simulink model "CdsnProbltyMgr"  (R2022b)
settings: Solver=FixedStepDiscrete  StartTime=0.0  StopTime=10.0  FixedStep=0.05  RelTol=1e-3
(part 1 of 2)
settings Model: Creator=blue.ge  LastModifiedBy=blue.ge
settings DataIOCC: SaveFormat=StructureWithTime
settings OptimizationCC: DefaultParameterBehavior=Inlined  EnhancedBackFolding=on  PassReuseOutputArgsAs=Individual arguments  ZeroExternalMemoryAtStartup=off  ZeroInternalMemoryAtStartup=off  OptimizeBlockOrder=speed  DifferentSizesBufferReuse=on  OptimizationCustomize=off  EfficientTunableParamExpr=on
settings DebuggingCC: ParameterTunabilityLossMsg=error
settings ModelReferenceCC: ModelReferenceNumInstancesAllowed=Single
settings SFSimCC: MATLABDynamicMemAlloc=off
settings RTWCC: Description=AUTOSAR  SystemTargetFile=autosar.tlc  GenCodeOnly=on  TemplateMakefile=ert_default_tmf
settings CodeAppCC: ForceParamTrailComments=on  IgnoreCustomStorageClasses=off  OperatorAnnotations=on  SimulinkDataObjDesc=on  SFDataObjDesc=on  InsertBlockDesc=on
settings STFCustomTargetCC: TargetLangStandard=C99 (ISO)  MultiwordLength=256  ParMdlRefBuildCompliant=on  CompOptLevelCompliant=on  IncludeMdlTerminateFcn=off  CombineOutputUpdateFcns=on  SuppressErrorStatus=on  ERTCustomFileBanners=on  SupportNonFinite=off  SupportComplex=off  SupportContinuousTime=off  SupportNonInlinedSFcns=off  RemoveResetFunc=on  MATLABClassNameForMDSCustomization=Simulink.SoftwareTarget.ERTCustomization  AutosarCompliant=on  UseToolchainInfoCompliant=on  LookupTableObjectStructAxisOrder=2,1,3,4,...  LUTObjectStructOrderEvenSpacing=Size,Table,Breakpoints  SystemTargetFile=autosar.tlc
settings ERTTargetCC: TargetLangStandard=C99 (ISO)  ConcurrentExecutionCompliant=off  IncludeMdlTerminateFcn=off  SuppressErrorStatus=on  SupportNonFinite=off  SupportComplex=off  ModelStepFunctionPrototypeControlCompliant=off  CPPClassGenCompliant=off  AutosarCompliant=on  LookupTableObjectStructAxisOrder=2,1,3,4,...  LUTObjectStructOrderEvenSpacing=Size,Table,Breakpoints  InlinedParameterPlacement=NonHierarchical  ERTCustomFileTemplate=example_file_process.tlc

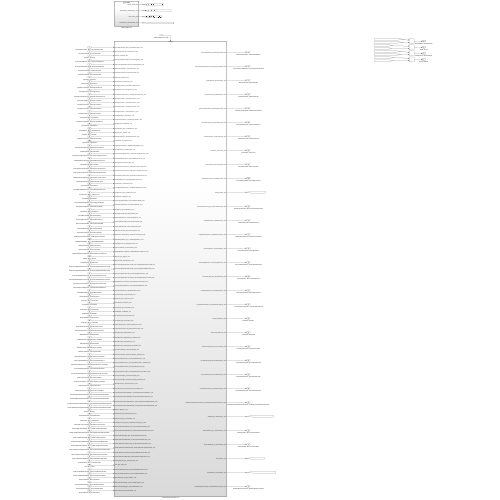
[thumbnail: Simulink's stored preview of the top-level diagram]
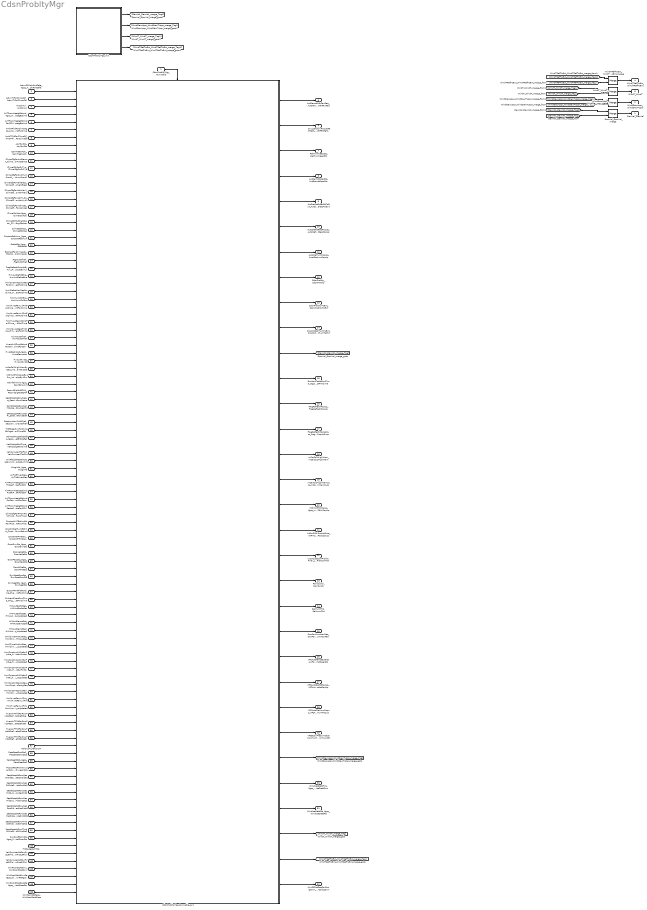
== system CdsnProbltyMgr ==
  CdsnProbltyMgr_Runnable : Inport  SampleTime=0.05  OutputFunctionCall=on
  ActvnOfWshrFrntSafe_Ngcc_ActvnOfWshrFrntSafe : Inport  Port=2  OutDataTypeStr=Enum: OnOffCrit1  PortDimensions=1
  ActvnTiForClimaCtrl_ActvnTiForClimaCtrl : Inport  Port=3  OutDataTypeStr=Ti33  PortDimensions=1
  AirCdnAvl_AirCdnAvl : Inport  Port=4  OutDataTypeStr=Boolean  PortDimensions=1
  AirTFromHeatgEstimd_Ngcc_AirTFromHeatgEstimd : Inport  Port=5  OutDataTypeStr=T30  PortDimensions=1
  AirTFromHeatgEstimdForCCM_AirTFromHeatgEstimd : Inport  Port=6  OutDataTypeStr=T30  PortDimensions=1
  AmbTFildForClimaNgcc_AmbTFildForClima : Inport  Port=7  OutDataTypeStr=T30  PortDimensions=1
  AmbTFildForClimaQf_AmbTFildForClimaQf : Inport  Port=8  OutDataTypeStr=Enum: GenQf1  PortDimensions=1
  AsyFanSts_AsyFanSts : Inport  Port=9  OutDataTypeStr=Enum: OnOff1  PortDimensions=1
  CamHeatrActv_CamHeatrActv : Inport  Port=10  OutDataTypeStr=Enum: OnOff1  PortDimensions=1
  ClimaCfgForAutDemst_ClimaCfgForAutDemst : Inport  Port=11  OutDataTypeStr=Boolean  PortDimensions=1
  ClimaCfgForFlcTyp_ClimaCfgForFlcTyp : Inport  Port=12  OutDataTypeStr=Enum: CarCfgForFlcTyp  PortDimensions=1
  ClimaCfgForSnsrHumPrsnt1_ClimaCfgForSnsrHumPrsnt1 : Inport  Port=13  OutDataTypeStr=Boolean  PortDimensions=1
  ClimaCfgForVehElec1_ClimaCfgForVehElec1 : Inport  Port=14  OutDataTypeStr=Boolean  PortDimensions=1
  ClimaCfgForVehHev1_ClimaCfgForVehHev1 : Inport  Port=15  OutDataTypeStr=Boolean  PortDimensions=1
  ClimaCfgForVehHyb1_ClimaCfgForVehHyb1 : Inport  Port=16  OutDataTypeStr=Boolean  PortDimensions=1
  ClimaCfgForVehIce1_ClimaCfgForVehIce1 : Inport  Port=17  OutDataTypeStr=Boolean  PortDimensions=1
  ClimaCtrlActvNgcc_ClimaCtrlActv : Inport  Port=18  OutDataTypeStr=Boolean  PortDimensions=1
  ClimaCtrlForEngStbActv_ClimaCtrlForEngStbActv : Inport  Port=19  OutDataTypeStr=Boolean  PortDimensions=1
  ClimaStStrtUp_ClimaStStrtUp : Inport  Port=20  OutDataTypeStr=Enum: ClimaStStrtUp  PortDimensions=1
  CmptmtRelHum_Ngcc_CmptmtRelHum : Inport  Port=21  OutDataTypeStr=Bus: CmptmRelHum  PortDimensions=1  BusOutputAsStruct=on
  DefrstSts_Ngcc_DefrstSts : Inport  Port=22  OutDataTypeStr=Bus: DefrstSts  PortDimensions=1  BusOutputAsStruct=on
  EcoModForClimaActv_EcoModForClimaActv : Inport  Port=23  OutDataTypeStr=Enum: OnOff1  PortDimensions=1
  FogOutOfVeh_FogOutOfVeh : Inport  Port=24  OutDataTypeStr=Bus: FogOutOfVeh  PortDimensions=1  BusOutputAsStruct=on
  FragRefreshPopUpByAux_FragRefreshPopUpByAux : Inport  Port=25  OutDataTypeStr=Enum: OnOff1  PortDimensions=1
  HmiAutDefrstEna_HmiAutDefrstEna : Inport  Port=26  OutDataTypeStr=Enum: OnOff1  PortDimensions=1
  HmiCmptmtCoolgReqForClima_HmiCmptmtCoolgReqForClima : Inport  Port=27  OutDataTypeStr=Enum: HmiCmptmtCoolgReq  PortDimensions=1
  HmiDefrstMaxReqForClima_HmiDefrstMaxReqForClima : Inport  Port=28  OutDataTypeStr=Enum: ActrReq  PortDimensions=1
  HmiHumCtrlEna_HmiHumCtrlEna : Inport  Port=29  OutDataTypeStr=Enum: OnOff1  PortDimensions=1
  HmiHvacFanLvlFrntForClima_HmiHvacFanLvlFrntForClima : Inport  Port=30  OutDataTypeStr=Enum: HmiHvacFanLvl  PortDimensions=1
  HmiHvacFanLvlFrntForClima_HmiHvacFanLvlFrntRiForClima : Inport  Port=31  OutDataTypeStr=Enum: HmiHvacFanLvl  PortDimensions=1
  HmiHvacRecircCmdForClima_HmiHvacRecircCmdForClima : Inport  Port=32  OutDataTypeStr=Enum: HmiHvacRecircCmd  PortDimensions=1
  HmiMaxACReqForClima_HmiMaxACReqForClima : Inport  Port=33  OutDataTypeStr=Enum: OnOff1  PortDimensions=1
  HumOutOfVeh_HumOutOfVeh : Inport  Port=34  OutDataTypeStr=Bus: HumOutOfVeh  PortDimensions=1  BusOutputAsStruct=on
  HvacAirMFlowEstimdForCtrlr_HvacAirMFlowEstimdForCtrlr : Inport  Port=35  OutDataTypeStr=AirFlow30  PortDimensions=1
  HvacRecircAct_Ngcc_HvacRecircAct : Inport  Port=36  OutDataTypeStr=Perc4  PortDimensions=1
  HvSysRlySts_HvSysRlySts : Inport  Port=37  OutDataTypeStr=Bus: HvSysRlySts  PortDimensions=1  BusOutputAsStruct=on
  InCarCo2HighWarnByCDS_InCarCo2HighWarnByCDS : Inport  Port=38  OutDataTypeStr=Enum: Co2Lvl  PortDimensions=1
  IntPm25HiPopUpByAPm_IntPm25HiPopUpByAPm : Inport  Port=39  OutDataTypeStr=Enum: IntPmHiPopUp  PortDimensions=1
  RainfallAmnt_Ngcc_RainfallAmnt : Inport  Port=40  OutDataTypeStr=Enum: AmntSnsr  PortDimensions=1
  ResrvdSigForECM4_ResrvdSigForECM4 : Inport  Port=41  OutDataTypeStr=BattSnsrChksTbl  PortDimensions=1
  SeatOccptAtRowSecLe_SeatOccptAtRowSecLe : Inport  Port=42  OutDataTypeStr=Enum: PassSeatSts1  PortDimensions=1
  SeatOccptAtRowSecMid_SeatOccptAtRowSecMid : Inport  Port=43  OutDataTypeStr=Enum: PassSeatSts1  PortDimensions=1
  SeatOccptAtRowSecRi_SeatOccptAtRowSecRi : Inport  Port=44  OutDataTypeStr=Enum: PassSeatSts1  PortDimensions=1
  SessionActvOutOfVeh_SessionActvOutOfVeh : Inport  Port=45  OutDataTypeStr=Enum: NoYes1  PortDimensions=1
  TiOfDeactvnForClimaCtrlNgcc_TiOfDeactvnForClimaCtrl : Inport  Port=46  OutDataTypeStr=Ti33  PortDimensions=1
  VehModMngtGlbSafe1_Ngcc_VehModMngtGlbSafe1 : Inport  Port=47  OutDataTypeStr=Bus: VehModMngtGlbSafe1  PortDimensions=1  BusOutputAsStruct=on
  VehSpdLgtForClima_VehSpdLgtForClima : Inport  Port=48  OutDataTypeStr=Bus: VehSpdLgtForClima  PortDimensions=1  BusOutputAsStruct=on
  VehSunLoadTotFild_VehSunLoadTotFild : Inport  Port=49  OutDataTypeStr=SunLoad30  PortDimensions=1
  WinRfClsdReqForCoolgDwnInt_WinRfClsdReqForCoolgDwnInt : Inport  Port=50  OutDataTypeStr=Enum: Warn2  PortDimensions=1
  WipgInfo_Ngcc_WipgInfo : Inport  Port=51  OutDataTypeStr=Bus: WipgInfo  PortDimensions=1  BusOutputAsStruct=on
  AirTAftHvacDes_AirTAftHvacDes : Inport  Port=52  OutDataTypeStr=T30  PortDimensions=1
  AirTFromHeatgEstimdFirstLeForCCM_AirTFromHeatgEstimdFirstLeForCCM : Inport  Port=53  OutDataTypeStr=T30  PortDimensions=1
  AirTFromHeatgEstimdFirstRiForCCM_AirTFromHeatgEstimdFirstRiForCCM : Inport  Port=54  OutDataTypeStr=T30  PortDimensions=1
  AirTFromHeatgEstimdForCdsn_AirTFromHeatgEstimdForCdsn : Inport  Port=55  OutDataTypeStr=T30  PortDimensions=1
  AirTFromHeatgEstimdSecLeForCCM_AirTFromHeatgEstimdSecLeForCCM : Inport  Port=56  OutDataTypeStr=T30  PortDimensions=1
  ClimaCfgForPTCInFrontHVAC_ClimaCfgForPTCInFrontHVAC : Inport  Port=57  OutDataTypeStr=Enum: FrontHeaterExchanger  PortDimensions=1
  CmptmtAirTEstimdAtRowFirst_CmptmtAirTEstimdAtRowFirst : Inport  Port=58  OutDataTypeStr=T30  PortDimensions=1
  CmptmtRelHumEstimd_CmptmtRelHumEstimd : Inport  Port=59  OutDataTypeStr=Perc7  PortDimensions=1
  CmptmtTFrntRaw_CmptmtTFrntRaw : Inport  Port=60  OutDataTypeStr=Bus: CmptmtTFrnt  PortDimensions=1  BusOutputAsStruct=on
  DoorDrvrSts_Ngcc_DoorDrvrSts : Inport  Port=61  OutDataTypeStr=Enum: DoorSts2  PortDimensions=1
  DoorLeReSts_DoorLeReSts : Inport  Port=62  OutDataTypeStr=Enum: DoorSts2  PortDimensions=1
  DoorPassSts_Ngcc_DoorPassSts : Inport  Port=63  OutDataTypeStr=Enum: DoorSts2  PortDimensions=1
  DoorRiReSts_DoorRiReSts : Inport  Port=64  OutDataTypeStr=Enum: DoorSts2  PortDimensions=1
  DrvrSeatSnsrSts_DrvrSeatSnsrSts : Inport  Port=65  OutDataTypeStr=Enum: OkNokDi  PortDimensions=1
  DrvrSeatSts_Ngcc_DrvrSeatSts : Inport  Port=66  OutDataTypeStr=Enum: OccptPresSt1  PortDimensions=1
  EvaprTFrntFildForClima_EvaprTFrntFildForClima : Inport  Port=67  OutDataTypeStr=T30  PortDimensions=1
  ExtVentToastFrmClima_Prkg_ExtVentToastFrmClima : Inport  Port=68  OutDataTypeStr=Enum: ExtVentToastFrmClimaSts  PortDimensions=1
  HMIAutDefrstSet_HMIAutDefrstSet : Inport  Port=69  OutDataTypeStr=Enum: OnOff1  PortDimensions=1
  HMIAutDefrstSet_HMIAutDefrstSet_IsUpdated : Inport  Port=70  OutDataTypeStr=boolean  PortDimensions=1
  HMIAutDemstSet_HMIAutDemstSet : Inport  Port=71  OutDataTypeStr=Enum: OnOff1  PortDimensions=1
  HMIAutDemstSet_HMIAutDemstSet_IsUpdated : Inport  Port=72  OutDataTypeStr=boolean  PortDimensions=1
  HmiClimaFrntAutReq_HmiClimaFrntAutReq : Inport  Port=73  OutDataTypeStr=Enum: OnOff1  PortDimensions=1
  HmiClimaFrntAutReq_HmiClimaFrntAutReq_IsUpdated : Inport  Port=74  OutDataTypeStr=boolean  PortDimensions=1
  HmiCmptmtAirDistbnFrntLe_HmiCmptmtAirDistbnFrntLe : Inport  Port=75  OutDataTypeStr=Enum: HmiCmptmtAirDistbnFrnt  PortDimensions=1
  HmiCmptmtAirDistbnFrntLe_HmiCmptmtAirDistbnFrntLe_IsUpdated : Inport  Port=76  OutDataTypeStr=boolean  PortDimensions=1
  HmiCmptmtAirDistbnFrntRi_HmiCmptmtAirDistbnFrntRi : Inport  Port=77  OutDataTypeStr=Enum: HmiCmptmtAirDistbnFrnt  PortDimensions=1
  HmiCmptmtAirDistbnFrntRi_HmiCmptmtAirDistbnFrntRi_IsUpdated : Inport  Port=78  OutDataTypeStr=boolean  PortDimensions=1
  HmiCmptmtCoolgReq_HmiCmptmtCoolgReq : Inport  Port=79  OutDataTypeStr=Enum: HmiCmptmtCoolgReq  PortDimensions=1
  HmiCmptmtCoolgReq_HmiCmptmtCoolgReq_IsUpdated : Inport  Port=80  OutDataTypeStr=boolean  PortDimensions=1
  HmiHvacFanLvlFrnt_HmiHvacFanLvlFrnt : Inport  Port=81  OutDataTypeStr=Enum: HmiHvacFanLvl  PortDimensions=1
  HmiHvacFanLvlFrnt_HmiHvacFanLvlFrnt_IsUpdated : Inport  Port=82  OutDataTypeStr=boolean  PortDimensions=1
  HvacAirTFildForRowFirstAtDefrstLe_HvacAirTFildForRowFirstAtDefrstLe : Inport  Port=83  OutDataTypeStr=T30  PortDimensions=1
  HvacAirTFildForRowFirstAtDefrstRi_HvacAirTFildForRowFirstAtDefrstRi : Inport  Port=84  OutDataTypeStr=T30  PortDimensions=1
  HvacAirTFildForRowFirstAtDefrstSideLe_HvacAirTFildForRowFirstAtDefrstSideLe : Inport  Port=85  OutDataTypeStr=T30  PortDimensions=1
  HvacAirTFildForRowFirstAtDefrstSideRi_HvacAirTFildForRowFirstAtDefrstSideRi : Inport  Port=86  OutDataTypeStr=T30  PortDimensions=1
  MtrlSnsrT_MtrlSnsrT : Inport  Port=87  OutDataTypeStr=Bus: MtrlSnsrT  PortDimensions=1  BusOutputAsStruct=on
  PassSeatSnsrSts2_PassSeatSnsrSts2 : Inport  Port=88  OutDataTypeStr=Enum: OkNokDi  PortDimensions=1
  PassSeatSts2_Ngcc_PassSeatSts2 : Inport  Port=89  OutDataTypeStr=Enum: OccptPresSt1  PortDimensions=1
  PopUpReqFromOxygenSply_PopUpReqFromOxygenSply : Inport  Port=90  OutDataTypeStr=Enum: OxygenSplyPopUpReq  PortDimensions=1
  SeatOccptAtRowSecDrSide2_Ngcc_SeatOccptAtRowSecDrSide2 : Inport  Port=91  OutDataTypeStr=Enum: OccptPresSt1  PortDimensions=1
  SeatOccptAtRowSecDrSideSnsSts2_SeatOccptAtRowSecDrSideSnsSts2 : Inport  Port=92  OutDataTypeStr=Enum: OkNokDi  PortDimensions=1
  SeatOccptAtRowSecMid2_Ngcc_SeatOccptAtRowSecMid2 : Inport  Port=93  OutDataTypeStr=Enum: OccptPresSt1  PortDimensions=1
  SeatOccptAtRowSecMidSnsSts2_SeatOccptAtRowSecMidSnsSts2 : Inport  Port=94  OutDataTypeStr=Enum: OkNokDi  PortDimensions=1
  SeatOccptAtRowSecPasSide2_Ngcc_SeatOccptAtRowSecPasSide2 : Inport  Port=95  OutDataTypeStr=Enum: OccptPresSt1  PortDimensions=1
  SeatOccptAtRowSecPasSideSnsSts2_SeatOccptAtRowSecPasSideSnsSts2 : Inport  Port=96  OutDataTypeStr=Enum: OkNokDi  PortDimensions=1
  SeatOccptAtRowThrdLeSnsSts2_SeatOccptAtRowThrdLeSnsSts2 : Inport  Port=97  OutDataTypeStr=Enum: OkNokDi  PortDimensions=1
  SeatOccptAtRowThrdRiSnsSts2_SeatOccptAtRowThrdRiSnsSts2 : Inport  Port=98  OutDataTypeStr=Enum: OkNokDi  PortDimensions=1
  SunRoofPosnSts_Ngcc_SunRoofPosnSts : Inport  Port=99  OutDataTypeStr=Enum: WinAndRoofAndCurtPosnTyp  PortDimensions=1
  TrSts_Ngcc_TrSts : Inport  Port=100  OutDataTypeStr=Enum: DoorSts2  PortDimensions=1
  VehSunLoadAtRowFirstLeFild_VehSunLoadAtRowFirstLeFild : Inport  Port=101  OutDataTypeStr=SunLoad30  PortDimensions=1
  VehSunLoadAtRowFirstRiFild_VehSunLoadAtRowFirstRiFild : Inport  Port=102  OutDataTypeStr=SunLoad30  PortDimensions=1
  WinPosnStsAtDrvr_WinPosnStsAtDrvr : Inport  Port=103  OutDataTypeStr=Enum: WinAndRoofAndCurtPosnTyp  PortDimensions=1
  WinPosnStsAtDrvrReNgcc_WinPosnStsAtDrvrReNgcc : Inport  Port=104  OutDataTypeStr=Enum: WinAndRoofAndCurtPosnTyp  PortDimensions=1
  WinPosnStsAtPassReNgcc_WinPosnStsAtPassRe : Inport  Port=105  OutDataTypeStr=Enum: WinAndRoofAndCurtPosnTyp  PortDimensions=1
  WinPosnStsPass_WinPosnStsAtPass : Inport  Port=106  OutDataTypeStr=Enum: WinAndRoofAndCurtPosnTyp  PortDimensions=1
  CdsnProbltyMgr_Init : Subsystem
  CdsnProbltyMgr_Runnable_sys : Subsystem
  DewVal_DewVal_merge : Merge
  WindDewUppr_WindDewTUppr_merge : Merge
  WindTRefToExt_WindTRefToExt_merge : Merge
  WindT_WindT_merge : Merge
  DewVal_DewVal : Outport  OutDataTypeStr=Bus: AirDewMeasValReq  PortDimensions=1  BusOutputAsStruct=on
  WindDewUppr_WindDewTUppr : Outport  Port=2  OutDataTypeStr=Bus: WindDewTUppr  PortDimensions=1  BusOutputAsStruct=on
  WindT_WindT : Outport  Port=3  OutDataTypeStr=Bus: WindT  PortDimensions=1  BusOutputAsStruct=on
  WindTRefToExt_WindTRefToExt : Outport  Port=4  OutDataTypeStr=Bus: WindT  PortDimensions=1  BusOutputAsStruct=on
  AutDemstWthFanReq_AutDemstWthFanReq : Outport  Port=5  OutDataTypeStr=UInt8  PortDimensions=1
  OxygenSplyPopUpReqNgcc_OxygenSplyPopUpReqNgcc : Outport  Port=6  OutDataTypeStr=Enum: OxygenSplyPopUpReq  PortDimensions=1
  RelHumKeepSts_RelHumKeepSts : Outport  Port=7  OutDataTypeStr=Enum: RelHumKeepSts  PortDimensions=1
  AutDemstOperSts_AutDemstOperSts : Outport  Port=8  OutDataTypeStr=Enum: OnOff1  PortDimensions=1
  AutDemstOperStsToExit_AutDemstOperStsToExit : Outport  Port=9  OutDataTypeStr=Enum: OnOff1  PortDimensions=1
  AutoDefrstReqPopUp_AutoDefrstReqPopUp : Outport  Port=10  OutDataTypeStr=Enum: OnOff1  PortDimensions=1
  AutoDeHumPopUp_AutoDeHumPopUp : Outport  Port=11  OutDataTypeStr=Enum: ReqSts1  PortDimensions=1
  CdsnProblty_CdsnProblty : Outport  Port=12  OutDataTypeStr=Fac30  PortDimensions=1
  CdsnProbltyToExt_CdsnProbltyToExt : Outport  Port=13  OutDataTypeStr=Fac30  PortDimensions=1
  CmptmtRelHumToExt_CmptmtRelHumToExt : Outport  Port=14  OutDataTypeStr=Bus: CmptmtRelHumToExt  PortDimensions=1  BusOutputAsStruct=on
  ExtVentToastFrmClima_Ngcc_ExtVentToastFrmClima : Outport  Port=15  OutDataTypeStr=Enum: ExtVentToastFrmClimaSts  PortDimensions=1
  FragRefreshPopUp_FragRefreshPopUp : Outport  Port=16  OutDataTypeStr=Enum: OnOff1  PortDimensions=1
  FragRefreshPopUpSucs_FragRefreshPopUpSucs : Outport  Port=17  OutDataTypeStr=Boolean  PortDimensions=1
  InCarCo2HighWarn_InCarCo2HighWarn : Outport  Port=18  OutDataTypeStr=Enum: Co2Lvl  PortDimensions=1
  InCarCo2HighWarnSucs_InCarCo2HighWarnSucs : Outport  Port=19  OutDataTypeStr=Boolean  PortDimensions=1
  IntPm25HiPopUp_Ngcc_IntPm25HiPopUp : Outport  Port=20  OutDataTypeStr=Enum: IntPmHiPopUp  PortDimensions=1
  IntPm25HiPopUpSucs_IntPm25HiPopUpSucs : Outport  Port=21  OutDataTypeStr=Boolean  PortDimensions=1
  LoopClsdCdsnProbltyFinal_LoopClsdCdsnProbltyFinal : Outport  Port=22  OutDataTypeStr=Fac30  PortDimensions=1
  RainSybol_RainSybol : Outport  Port=23  OutDataTypeStr=Boolean  PortDimensions=1
  RelHumFild_RelHumFild : Outport  Port=24  OutDataTypeStr=Perc7  PortDimensions=1
  SnsrForHumActvReq_SnsrForHumActvReq : Outport  Port=25  OutDataTypeStr=Enum: OnOff1  PortDimensions=1
  VFCAutDemstOperSts_VFCAutDemstOperSts : Outport  Port=26  OutDataTypeStr=Boolean  PortDimensions=1
  VFCAutoDefrstPopUp_VFCAutoDefrstPopUp : Outport  Port=27  OutDataTypeStr=Boolean  PortDimensions=1
  VFCAutoDeHumPopup_VFCAutoDeHumPopup : Outport  Port=28  OutDataTypeStr=Boolean  PortDimensions=1
  VFCBodyPreClimatisationHmiHumCtrl_VFCBodyPreClimatisationHmiHumCtrl : Outport  Port=29  OutDataTypeStr=Boolean  PortDimensions=1
  WindIceDetdFrnt_Ngcc_WindIceDetdFrnt : Outport  Port=30  OutDataTypeStr=Enum: OnOff1  PortDimensions=1
  WindIceDetdRe_Ngcc_WindIceDetdRe : Outport  Port=31  OutDataTypeStr=Enum: OnOff1  PortDimensions=1
  WinRfClsdReqForCoolgDwn_WinRfClsdReqForCoolgDwn : Outport  Port=32  OutDataTypeStr=Enum: Warn2  PortDimensions=1
  wires:
  ActvnOfWshrFrntSafe_Ngcc_ActvnOfWshrFrntSafe/1 -> CdsnProbltyMgr_Runnable_sys/1(ActvnOfWshrFrntSafe_Ngcc_ActvnOfWshrFrntSafe_read)
  ActvnTiForClimaCtrl_ActvnTiForClimaCtrl/1 -> CdsnProbltyMgr_Runnable_sys/2(ActvnTiForClimaCtrl_ActvnTiForClimaCtrl_read)
  AirCdnAvl_AirCdnAvl/1 -> CdsnProbltyMgr_Runnable_sys/3(AirCdnAvl_AirCdnAvl_read)
  AirTFromHeatgEstimd_Ngcc_AirTFromHeatgEstimd/1 -> CdsnProbltyMgr_Runnable_sys/4(AirTFromHeatgEstimd_Ngcc_AirTFromHeatgEstimd_read)
  AirTFromHeatgEstimdForCCM_AirTFromHeatgEstimd/1 -> CdsnProbltyMgr_Runnable_sys/5(AirTFromHeatgEstimdForCCM_AirTFromHeatgEstimd_read)
  AmbTFildForClimaNgcc_AmbTFildForClima/1 -> CdsnProbltyMgr_Runnable_sys/6(AmbTFildForClimaNgcc_AmbTFildForClima_read)
  AmbTFildForClimaQf_AmbTFildForClimaQf/1 -> CdsnProbltyMgr_Runnable_sys/7(AmbTFildForClimaQf_AmbTFildForClimaQf_read)
  AsyFanSts_AsyFanSts/1 -> CdsnProbltyMgr_Runnable_sys/8(AsyFanSts_AsyFanSts_read)
  CamHeatrActv_CamHeatrActv/1 -> CdsnProbltyMgr_Runnable_sys/9(CamHeatrActv_CamHeatrActv_read)
  ClimaCfgForAutDemst_ClimaCfgForAutDemst/1 -> CdsnProbltyMgr_Runnable_sys/10(ClimaCfgForAutDemst_ClimaCfgForAutDemst_read)
  ClimaCfgForFlcTyp_ClimaCfgForFlcTyp/1 -> CdsnProbltyMgr_Runnable_sys/11(ClimaCfgForFlcTyp_ClimaCfgForFlcTyp_read)
  ClimaCfgForSnsrHumPrsnt1_ClimaCfgForSnsrHumPrsnt1/1 -> CdsnProbltyMgr_Runnable_sys/12(ClimaCfgForSnsrHumPrsnt1_ClimaCfgForSnsrHumPrsnt1_read)
  ClimaCfgForVehElec1_ClimaCfgForVehElec1/1 -> CdsnProbltyMgr_Runnable_sys/13(ClimaCfgForVehElec1_ClimaCfgForVehElec1_read)
  ClimaCfgForVehHev1_ClimaCfgForVehHev1/1 -> CdsnProbltyMgr_Runnable_sys/14(ClimaCfgForVehHev1_ClimaCfgForVehHev1_read)
  ClimaCfgForVehHyb1_ClimaCfgForVehHyb1/1 -> CdsnProbltyMgr_Runnable_sys/15(ClimaCfgForVehHyb1_ClimaCfgForVehHyb1_read)
  ClimaCfgForVehIce1_ClimaCfgForVehIce1/1 -> CdsnProbltyMgr_Runnable_sys/16(ClimaCfgForVehIce1_ClimaCfgForVehIce1_read)
  ClimaCtrlActvNgcc_ClimaCtrlActv/1 -> CdsnProbltyMgr_Runnable_sys/17(ClimaCtrlActvNgcc_ClimaCtrlActv_read)
  ClimaCtrlForEngStbActv_ClimaCtrlForEngStbActv/1 -> CdsnProbltyMgr_Runnable_sys/18(ClimaCtrlForEngStbActv_ClimaCtrlForEngStbActv_read)
  ClimaStStrtUp_ClimaStStrtUp/1 -> CdsnProbltyMgr_Runnable_sys/19(ClimaStStrtUp_ClimaStStrtUp_read)
  CmptmtRelHum_Ngcc_CmptmtRelHum/1 -> CdsnProbltyMgr_Runnable_sys/20(CmptmtRelHum_Ngcc_CmptmtRelHum_read)
  DefrstSts_Ngcc_DefrstSts/1 -> CdsnProbltyMgr_Runnable_sys/21(DefrstSts_Ngcc_DefrstSts_read)
  EcoModForClimaActv_EcoModForClimaActv/1 -> CdsnProbltyMgr_Runnable_sys/22(EcoModForClimaActv_EcoModForClimaActv_read)
  FogOutOfVeh_FogOutOfVeh/1 -> CdsnProbltyMgr_Runnable_sys/23(FogOutOfVeh_FogOutOfVeh_read)
  FragRefreshPopUpByAux_FragRefreshPopUpByAux/1 -> CdsnProbltyMgr_Runnable_sys/24(FragRefreshPopUpByAux_FragRefreshPopUpByAux_read)
  HmiAutDefrstEna_HmiAutDefrstEna/1 -> CdsnProbltyMgr_Runnable_sys/25(HmiAutDefrstEna_HmiAutDefrstEna_read)
  HmiCmptmtCoolgReqForClima_HmiCmptmtCoolgReqForClima/1 -> CdsnProbltyMgr_Runnable_sys/26(HmiCmptmtCoolgReqForClima_HmiCmptmtCoolgReqForClima_read)
  HmiDefrstMaxReqForClima_HmiDefrstMaxReqForClima/1 -> CdsnProbltyMgr_Runnable_sys/27(HmiDefrstMaxReqForClima_HmiDefrstMaxReqForClima_read)
  HmiHumCtrlEna_HmiHumCtrlEna/1 -> CdsnProbltyMgr_Runnable_sys/28(HmiHumCtrlEna_HmiHumCtrlEna_read)
  HmiHvacFanLvlFrntForClima_HmiHvacFanLvlFrntForClima/1 -> CdsnProbltyMgr_Runnable_sys/29(HmiHvacFanLvlFrntForClima_HmiHvacFanLvlFrntForClima_read)
  HmiHvacFanLvlFrntForClima_HmiHvacFanLvlFrntRiForClima/1 -> CdsnProbltyMgr_Runnable_sys/30(HmiHvacFanLvlFrntForClima_HmiHvacFanLvlFrntRiForClima_read)
  HmiHvacRecircCmdForClima_HmiHvacRecircCmdForClima/1 -> CdsnProbltyMgr_Runnable_sys/31(HmiHvacRecircCmdForClima_HmiHvacRecircCmdForClima_read)
  HmiMaxACReqForClima_HmiMaxACReqForClima/1 -> CdsnProbltyMgr_Runnable_sys/32(HmiMaxACReqForClima_HmiMaxACReqForClima_read)
  HumOutOfVeh_HumOutOfVeh/1 -> CdsnProbltyMgr_Runnable_sys/33(HumOutOfVeh_HumOutOfVeh_read)
  HvacAirMFlowEstimdForCtrlr_HvacAirMFlowEstimdForCtrlr/1 -> CdsnProbltyMgr_Runnable_sys/34(HvacAirMFlowEstimdForCtrlr_HvacAirMFlowEstimdForCtrlr_read)
  HvacRecircAct_Ngcc_HvacRecircAct/1 -> CdsnProbltyMgr_Runnable_sys/35(HvacRecircAct_Ngcc_HvacRecircAct_read)
  HvSysRlySts_HvSysRlySts/1 -> CdsnProbltyMgr_Runnable_sys/36(HvSysRlySts_HvSysRlySts_read)
  InCarCo2HighWarnByCDS_InCarCo2HighWarnByCDS/1 -> CdsnProbltyMgr_Runnable_sys/37(InCarCo2HighWarnByCDS_InCarCo2HighWarnByCDS_read)
  IntPm25HiPopUpByAPm_IntPm25HiPopUpByAPm/1 -> CdsnProbltyMgr_Runnable_sys/38(IntPm25HiPopUpByAPm_IntPm25HiPopUpByAPm_read)
  RainfallAmnt_Ngcc_RainfallAmnt/1 -> CdsnProbltyMgr_Runnable_sys/39(RainfallAmnt_Ngcc_RainfallAmnt_read)
  ResrvdSigForECM4_ResrvdSigForECM4/1 -> CdsnProbltyMgr_Runnable_sys/40(ResrvdSigForECM4_ResrvdSigForECM4_read)
  SeatOccptAtRowSecLe_SeatOccptAtRowSecLe/1 -> CdsnProbltyMgr_Runnable_sys/41(SeatOccptAtRowSecLe_SeatOccptAtRowSecLe_read)
  SeatOccptAtRowSecMid_SeatOccptAtRowSecMid/1 -> CdsnProbltyMgr_Runnable_sys/42(SeatOccptAtRowSecMid_SeatOccptAtRowSecMid_read)
  SeatOccptAtRowSecRi_SeatOccptAtRowSecRi/1 -> CdsnProbltyMgr_Runnable_sys/43(SeatOccptAtRowSecRi_SeatOccptAtRowSecRi_read)
  SessionActvOutOfVeh_SessionActvOutOfVeh/1 -> CdsnProbltyMgr_Runnable_sys/44(SessionActvOutOfVeh_SessionActvOutOfVeh_read)
  TiOfDeactvnForClimaCtrlNgcc_TiOfDeactvnForClimaCtrl/1 -> CdsnProbltyMgr_Runnable_sys/45(TiOfDeactvnForClimaCtrlNgcc_TiOfDeactvnForClimaCtrl_read)
  VehModMngtGlbSafe1_Ngcc_VehModMngtGlbSafe1/1 -> CdsnProbltyMgr_Runnable_sys/46(VehModMngtGlbSafe1_Ngcc_VehModMngtGlbSafe1_read)
  VehSpdLgtForClima_VehSpdLgtForClima/1 -> CdsnProbltyMgr_Runnable_sys/47(VehSpdLgtForClima_VehSpdLgtForClima_read)
  VehSunLoadTotFild_VehSunLoadTotFild/1 -> CdsnProbltyMgr_Runnable_sys/48(VehSunLoadTotFild_VehSunLoadTotFild_read)
  WinRfClsdReqForCoolgDwnInt_WinRfClsdReqForCoolgDwnInt/1 -> CdsnProbltyMgr_Runnable_sys/49(WinRfClsdReqForCoolgDwnInt_WinRfClsdReqForCoolgDwnInt_read)
  WipgInfo_Ngcc_WipgInfo/1 -> CdsnProbltyMgr_Runnable_sys/50(WipgInfo_Ngcc_WipgInfo_read)
  AirTAftHvacDes_AirTAftHvacDes/1 -> CdsnProbltyMgr_Runnable_sys/51(AirTAftHvacDes_AirTAftHvacDes_read)
  AirTFromHeatgEstimdFirstLeForCCM_AirTFromHeatgEstimdFirstLeForCCM/1 -> CdsnProbltyMgr_Runnable_sys/52(AirTFromHeatgEstimdFirstLeForCCM_AirTFromHeatgEstimdFirstLeForCCM_read)
  AirTFromHeatgEstimdFirstRiForCCM_AirTFromHeatgEstimdFirstRiForCCM/1 -> CdsnProbltyMgr_Runnable_sys/53(AirTFromHeatgEstimdFirstRiForCCM_AirTFromHeatgEstimdFirstRiForCCM_read)
  AirTFromHeatgEstimdForCdsn_AirTFromHeatgEstimdForCdsn/1 -> CdsnProbltyMgr_Runnable_sys/54(AirTFromHeatgEstimdForCdsn_AirTFromHeatgEstimdForCdsn_read)
  AirTFromHeatgEstimdSecLeForCCM_AirTFromHeatgEstimdSecLeForCCM/1 -> CdsnProbltyMgr_Runnable_sys/55(AirTFromHeatgEstimdSecLeForCCM_AirTFromHeatgEstimdSecLeForCCM_read)
  ClimaCfgForPTCInFrontHVAC_ClimaCfgForPTCInFrontHVAC/1 -> CdsnProbltyMgr_Runnable_sys/56(ClimaCfgForPTCInFrontHVAC_ClimaCfgForPTCInFrontHVAC_read)
  CmptmtAirTEstimdAtRowFirst_CmptmtAirTEstimdAtRowFirst/1 -> CdsnProbltyMgr_Runnable_sys/57(CmptmtAirTEstimdAtRowFirst_CmptmtAirTEstimdAtRowFirst_read)
  CmptmtRelHumEstimd_CmptmtRelHumEstimd/1 -> CdsnProbltyMgr_Runnable_sys/58(CmptmtRelHumEstimd_CmptmtRelHumEstimd_read)
  CmptmtTFrntRaw_CmptmtTFrntRaw/1 -> CdsnProbltyMgr_Runnable_sys/59(CmptmtTFrntRaw_CmptmtTFrntRaw_read)
  DoorDrvrSts_Ngcc_DoorDrvrSts/1 -> CdsnProbltyMgr_Runnable_sys/60(DoorDrvrSts_Ngcc_DoorDrvrSts_read)
  DoorLeReSts_DoorLeReSts/1 -> CdsnProbltyMgr_Runnable_sys/61(DoorLeReSts_DoorLeReSts_read)
  DoorPassSts_Ngcc_DoorPassSts/1 -> CdsnProbltyMgr_Runnable_sys/62(DoorPassSts_Ngcc_DoorPassSts_read)
  DoorRiReSts_DoorRiReSts/1 -> CdsnProbltyMgr_Runnable_sys/63(DoorRiReSts_DoorRiReSts_read)
  DrvrSeatSnsrSts_DrvrSeatSnsrSts/1 -> CdsnProbltyMgr_Runnable_sys/64(DrvrSeatSnsrSts_DrvrSeatSnsrSts_read)
  DrvrSeatSts_Ngcc_DrvrSeatSts/1 -> CdsnProbltyMgr_Runnable_sys/65(DrvrSeatSts_Ngcc_DrvrSeatSts_read)
  EvaprTFrntFildForClima_EvaprTFrntFildForClima/1 -> CdsnProbltyMgr_Runnable_sys/66(EvaprTFrntFildForClima_EvaprTFrntFildForClima_read)
  ExtVentToastFrmClima_Prkg_ExtVentToastFrmClima/1 -> CdsnProbltyMgr_Runnable_sys/67(ExtVentToastFrmClima_Prkg_ExtVentToastFrmClima_read)
  HMIAutDefrstSet_HMIAutDefrstSet/1 -> CdsnProbltyMgr_Runnable_sys/68(HMIAutDefrstSet_HMIAutDefrstSet_read)
  HMIAutDefrstSet_HMIAutDefrstSet_IsUpdated/1 -> CdsnProbltyMgr_Runnable_sys/69(HMIAutDefrstSet_HMIAutDefrstSet_IsUpdated_read)
  HMIAutDemstSet_HMIAutDemstSet/1 -> CdsnProbltyMgr_Runnable_sys/70(HMIAutDemstSet_HMIAutDemstSet_read)
  HMIAutDemstSet_HMIAutDemstSet_IsUpdated/1 -> CdsnProbltyMgr_Runnable_sys/71(HMIAutDemstSet_HMIAutDemstSet_IsUpdated_read)
  HmiClimaFrntAutReq_HmiClimaFrntAutReq/1 -> CdsnProbltyMgr_Runnable_sys/72(HmiClimaFrntAutReq_HmiClimaFrntAutReq_read)
  HmiClimaFrntAutReq_HmiClimaFrntAutReq_IsUpdated/1 -> CdsnProbltyMgr_Runnable_sys/73(HmiClimaFrntAutReq_HmiClimaFrntAutReq_IsUpdated_read)
  HmiCmptmtAirDistbnFrntLe_HmiCmptmtAirDistbnFrntLe/1 -> CdsnProbltyMgr_Runnable_sys/74(HmiCmptmtAirDistbnFrntLe_HmiCmptmtAirDistbnFrntLe_read)
  HmiCmptmtAirDistbnFrntLe_HmiCmptmtAirDistbnFrntLe_IsUpdated/1 -> CdsnProbltyMgr_Runnable_sys/75(HmiCmptmtAirDistbnFrntLe_HmiCmptmtAirDistbnFrntLe_IsUpdated_read)
  HmiCmptmtAirDistbnFrntRi_HmiCmptmtAirDistbnFrntRi/1 -> CdsnProbltyMgr_Runnable_sys/76(HmiCmptmtAirDistbnFrntRi_HmiCmptmtAirDistbnFrntRi_read)
  HmiCmptmtAirDistbnFrntRi_HmiCmptmtAirDistbnFrntRi_IsUpdated/1 -> CdsnProbltyMgr_Runnable_sys/77(HmiCmptmtAirDistbnFrntRi_HmiCmptmtAirDistbnFrntRi_IsUpdated_read)
  HmiCmptmtCoolgReq_HmiCmptmtCoolgReq/1 -> CdsnProbltyMgr_Runnable_sys/78(HmiCmptmtCoolgReq_HmiCmptmtCoolgReq_read)
  HmiCmptmtCoolgReq_HmiCmptmtCoolgReq_IsUpdated/1 -> CdsnProbltyMgr_Runnable_sys/79(HmiCmptmtCoolgReq_HmiCmptmtCoolgReq_IsUpdated_read)
  HmiHvacFanLvlFrnt_HmiHvacFanLvlFrnt/1 -> CdsnProbltyMgr_Runnable_sys/80(HmiHvacFanLvlFrnt_HmiHvacFanLvlFrnt_read)
  HmiHvacFanLvlFrnt_HmiHvacFanLvlFrnt_IsUpdated/1 -> CdsnProbltyMgr_Runnable_sys/81(HmiHvacFanLvlFrnt_HmiHvacFanLvlFrnt_IsUpdated_read)
  HvacAirTFildForRowFirstAtDefrstLe_HvacAirTFildForRowFirstAtDefrstLe/1 -> CdsnProbltyMgr_Runnable_sys/82(HvacAirTFildForRowFirstAtDefrstLe_HvacAirTFildForRowFirstAtDefrstLe_read)
  HvacAirTFildForRowFirstAtDefrstRi_HvacAirTFildForRowFirstAtDefrstRi/1 -> CdsnProbltyMgr_Runnable_sys/83(HvacAirTFildForRowFirstAtDefrstRi_HvacAirTFildForRowFirstAtDefrstRi_read)
  HvacAirTFildForRowFirstAtDefrstSideLe_HvacAirTFildForRowFirstAtDefrstSideLe/1 -> CdsnProbltyMgr_Runnable_sys/84(HvacAirTFildForRowFirstAtDefrstSideLe_HvacAirTFildForRowFirstAtDefrstSideLe_read)
  HvacAirTFildForRowFirstAtDefrstSideRi_HvacAirTFildForRowFirstAtDefrstSideRi/1 -> CdsnProbltyMgr_Runnable_sys/85(HvacAirTFildForRowFirstAtDefrstSideRi_HvacAirTFildForRowFirstAtDefrstSideRi_read)
  MtrlSnsrT_MtrlSnsrT/1 -> CdsnProbltyMgr_Runnable_sys/86(MtrlSnsrT_MtrlSnsrT_read)
  PassSeatSnsrSts2_PassSeatSnsrSts2/1 -> CdsnProbltyMgr_Runnable_sys/87(PassSeatSnsrSts2_PassSeatSnsrSts2_read)
  PassSeatSts2_Ngcc_PassSeatSts2/1 -> CdsnProbltyMgr_Runnable_sys/88(PassSeatSts2_Ngcc_PassSeatSts2_read)
  PopUpReqFromOxygenSply_PopUpReqFromOxygenSply/1 -> CdsnProbltyMgr_Runnable_sys/89(PopUpReqFromOxygenSply_PopUpReqFromOxygenSply_read)
  SeatOccptAtRowSecDrSide2_Ngcc_SeatOccptAtRowSecDrSide2/1 -> CdsnProbltyMgr_Runnable_sys/90(SeatOccptAtRowSecDrSide2_Ngcc_SeatOccptAtRowSecDrSide2_read)
  SeatOccptAtRowSecDrSideSnsSts2_SeatOccptAtRowSecDrSideSnsSts2/1 -> CdsnProbltyMgr_Runnable_sys/91(SeatOccptAtRowSecDrSideSnsSts2_SeatOccptAtRowSecDrSideSnsSts2_read)
  SeatOccptAtRowSecMid2_Ngcc_SeatOccptAtRowSecMid2/1 -> CdsnProbltyMgr_Runnable_sys/92(SeatOccptAtRowSecMid2_Ngcc_SeatOccptAtRowSecMid2_read)
  SeatOccptAtRowSecMidSnsSts2_SeatOccptAtRowSecMidSnsSts2/1 -> CdsnProbltyMgr_Runnable_sys/93(SeatOccptAtRowSecMidSnsSts2_SeatOccptAtRowSecMidSnsSts2_read)
  SeatOccptAtRowSecPasSide2_Ngcc_SeatOccptAtRowSecPasSide2/1 -> CdsnProbltyMgr_Runnable_sys/94(SeatOccptAtRowSecPasSide2_Ngcc_SeatOccptAtRowSecPasSide2_read)
  SeatOccptAtRowSecPasSideSnsSts2_SeatOccptAtRowSecPasSideSnsSts2/1 -> CdsnProbltyMgr_Runnable_sys/95(SeatOccptAtRowSecPasSideSnsSts2_SeatOccptAtRowSecPasSideSnsSts2_read)
  SeatOccptAtRowThrdLeSnsSts2_SeatOccptAtRowThrdLeSnsSts2/1 -> CdsnProbltyMgr_Runnable_sys/96(SeatOccptAtRowThrdLeSnsSts2_SeatOccptAtRowThrdLeSnsSts2_read)
  SeatOccptAtRowThrdRiSnsSts2_SeatOccptAtRowThrdRiSnsSts2/1 -> CdsnProbltyMgr_Runnable_sys/97(SeatOccptAtRowThrdRiSnsSts2_SeatOccptAtRowThrdRiSnsSts2_read)
  SunRoofPosnSts_Ngcc_SunRoofPosnSts/1 -> CdsnProbltyMgr_Runnable_sys/98(SunRoofPosnSts_Ngcc_SunRoofPosnSts_read)
  TrSts_Ngcc_TrSts/1 -> CdsnProbltyMgr_Runnable_sys/99(TrSts_Ngcc_TrSts_read)
  VehSunLoadAtRowFirstLeFild_VehSunLoadAtRowFirstLeFild/1 -> CdsnProbltyMgr_Runnable_sys/100(VehSunLoadAtRowFirstLeFild_VehSunLoadAtRowFirstLeFild_read)
  VehSunLoadAtRowFirstRiFild_VehSunLoadAtRowFirstRiFild/1 -> CdsnProbltyMgr_Runnable_sys/101(VehSunLoadAtRowFirstRiFild_VehSunLoadAtRowFirstRiFild_read)
  WinPosnStsAtDrvr_WinPosnStsAtDrvr/1 -> CdsnProbltyMgr_Runnable_sys/102(WinPosnStsAtDrvr_WinPosnStsAtDrvr_read)
  WinPosnStsAtDrvrReNgcc_WinPosnStsAtDrvrReNgcc/1 -> CdsnProbltyMgr_Runnable_sys/103(WinPosnStsAtDrvrReNgcc_WinPosnStsAtDrvrReNgcc_read)
  WinPosnStsAtPassReNgcc_WinPosnStsAtPassRe/1 -> CdsnProbltyMgr_Runnable_sys/104(WinPosnStsAtPassReNgcc_WinPosnStsAtPassRe_read)
  WinPosnStsPass_WinPosnStsAtPass/1 -> CdsnProbltyMgr_Runnable_sys/105(WinPosnStsPass_WinPosnStsAtPass_read)
  CdsnProbltyMgr_Runnable_sys/1(AutDemstWthFanReq_AutDemstWthFanReq_write) -> AutDemstWthFanReq_AutDemstWthFanReq/1
  CdsnProbltyMgr_Runnable_sys/2(OxygenSplyPopUpReqNgcc_OxygenSplyPopUpReqNgcc_write) -> OxygenSplyPopUpReqNgcc_OxygenSplyPopUpReqNgcc/1
  CdsnProbltyMgr_Runnable_sys/3(RelHumKeepSts_RelHumKeepSts_write) -> RelHumKeepSts_RelHumKeepSts/1
  CdsnProbltyMgr_Runnable_sys/4(AutDemstOperSts_AutDemstOperSts_write) -> AutDemstOperSts_AutDemstOperSts/1
  CdsnProbltyMgr_Runnable_sys/5(AutDemstOperStsToExit_AutDemstOperStsToExit_write) -> AutDemstOperStsToExit_AutDemstOperStsToExit/1
  CdsnProbltyMgr_Runnable_sys/6(AutoDefrstReqPopUp_AutoDefrstReqPopUp_write) -> AutoDefrstReqPopUp_AutoDefrstReqPopUp/1
  CdsnProbltyMgr_Runnable_sys/7(AutoDeHumPopUp_AutoDeHumPopUp_write) -> AutoDeHumPopUp_AutoDeHumPopUp/1
  CdsnProbltyMgr_Runnable_sys/8(CdsnProblty_CdsnProblty_write) -> CdsnProblty_CdsnProblty/1
  CdsnProbltyMgr_Runnable_sys/9(CdsnProbltyToExt_CdsnProbltyToExt_write) -> CdsnProbltyToExt_CdsnProbltyToExt/1
  CdsnProbltyMgr_Runnable_sys/10(CmptmtRelHumToExt_CmptmtRelHumToExt_write) -> CmptmtRelHumToExt_CmptmtRelHumToExt/1
  DewVal_DewVal_merge/1 -> DewVal_DewVal/1
  CdsnProbltyMgr_Runnable_sys/12(ExtVentToastFrmClima_Ngcc_ExtVentToastFrmClima_write) -> ExtVentToastFrmClima_Ngcc_ExtVentToastFrmClima/1
  CdsnProbltyMgr_Runnable_sys/13(FragRefreshPopUp_FragRefreshPopUp_write) -> FragRefreshPopUp_FragRefreshPopUp/1
  CdsnProbltyMgr_Runnable_sys/14(FragRefreshPopUpSucs_FragRefreshPopUpSucs_write) -> FragRefreshPopUpSucs_FragRefreshPopUpSucs/1
  CdsnProbltyMgr_Runnable_sys/15(InCarCo2HighWarn_InCarCo2HighWarn_write) -> InCarCo2HighWarn_InCarCo2HighWarn/1
  CdsnProbltyMgr_Runnable_sys/16(InCarCo2HighWarnSucs_InCarCo2HighWarnSucs_write) -> InCarCo2HighWarnSucs_InCarCo2HighWarnSucs/1
  CdsnProbltyMgr_Runnable_sys/17(IntPm25HiPopUp_Ngcc_IntPm25HiPopUp_write) -> IntPm25HiPopUp_Ngcc_IntPm25HiPopUp/1
  CdsnProbltyMgr_Runnable_sys/18(IntPm25HiPopUpSucs_IntPm25HiPopUpSucs_write) -> IntPm25HiPopUpSucs_IntPm25HiPopUpSucs/1
  CdsnProbltyMgr_Runnable_sys/19(LoopClsdCdsnProbltyFinal_LoopClsdCdsnProbltyFinal_write) -> LoopClsdCdsnProbltyFinal_LoopClsdCdsnProbltyFinal/1
  CdsnProbltyMgr_Runnable_sys/20(RainSybol_RainSybol_write) -> RainSybol_RainSybol/1
  CdsnProbltyMgr_Runnable_sys/21(RelHumFild_RelHumFild_write) -> RelHumFild_RelHumFild/1
  CdsnProbltyMgr_Runnable_sys/22(SnsrForHumActvReq_SnsrForHumActvReq_write) -> SnsrForHumActvReq_SnsrForHumActvReq/1
  CdsnProbltyMgr_Runnable_sys/23(VFCAutDemstOperSts_VFCAutDemstOperSts_write) -> VFCAutDemstOperSts_VFCAutDemstOperSts/1
  CdsnProbltyMgr_Runnable_sys/24(VFCAutoDefrstPopUp_VFCAutoDefrstPopUp_write) -> VFCAutoDefrstPopUp_VFCAutoDefrstPopUp/1
  CdsnProbltyMgr_Runnable_sys/25(VFCAutoDeHumPopup_VFCAutoDeHumPopup_write) -> VFCAutoDeHumPopup_VFCAutoDeHumPopup/1
  CdsnProbltyMgr_Runnable_sys/26(VFCBodyPreClimatisationHmiHumCtrl_VFCBodyPreClimatisationHmiHumCtrl_write) -> VFCBodyPreClimatisationHmiHumCtrl_VFCBodyPreClimatisationHmiHumCtrl/1
  WindDewUppr_WindDewTUppr_merge/1 -> WindDewUppr_WindDewTUppr/1
  CdsnProbltyMgr_Runnable_sys/28(WindIceDetdFrnt_Ngcc_WindIceDetdFrnt_write) -> WindIceDetdFrnt_Ngcc_WindIceDetdFrnt/1
  CdsnProbltyMgr_Runnable_sys/29(WindIceDetdRe_Ngcc_WindIceDetdRe_write) -> WindIceDetdRe_Ngcc_WindIceDetdRe/1
  WindT_WindT_merge/1 -> WindT_WindT/1
  WindTRefToExt_WindTRefToExt_merge/1 -> WindTRefToExt_WindTRefToExt/1
  CdsnProbltyMgr_Runnable_sys/32(WinRfClsdReqForCoolgDwn_WinRfClsdReqForCoolgDwn_write) -> WinRfClsdReqForCoolgDwn_WinRfClsdReqForCoolgDwn/1
  CdsnProbltyMgr_Runnable_sys/31(WindTRefToExt_WindTRefToExt_write) -> [WindTRefToExt_WindTRefToExt_merge_Tag]
  [WindTRefToExt_WindTRefToExt_merge_Tag] -> WindTRefToExt_WindTRefToExt_merge/2
  CdsnProbltyMgr_Init/4(WindTRefToExt_WindTRefToExt_write) -> [WindTRefToExt_WindTRefToExt_merge_Tag1]
  [WindTRefToExt_WindTRefToExt_merge_Tag1] -> WindTRefToExt_WindTRefToExt_merge/1
  CdsnProbltyMgr_Runnable_sys/30(WindT_WindT_write) -> [WindT_WindT_merge_Tag]
  [WindT_WindT_merge_Tag] -> WindT_WindT_merge/2
  CdsnProbltyMgr_Init/3(WindT_WindT_write) -> [WindT_WindT_merge_Tag1]
  [WindT_WindT_merge_Tag1] -> WindT_WindT_merge/1
  CdsnProbltyMgr_Runnable_sys/27(WindDewUppr_WindDewTUppr_write) -> [WindDewUppr_WindDewTUppr_merge_Tag]
  [WindDewUppr_WindDewTUppr_merge_Tag] -> WindDewUppr_WindDewTUppr_merge/2
  CdsnProbltyMgr_Init/2(WindDewUppr_WindDewTUppr_write) -> [WindDewUppr_WindDewTUppr_merge_Tag1]
  [WindDewUppr_WindDewTUppr_merge_Tag1] -> WindDewUppr_WindDewTUppr_merge/1
  CdsnProbltyMgr_Runnable_sys/11(DewVal_DewVal_write) -> [DewVal_DewVal_merge_Tag]
  [DewVal_DewVal_merge_Tag] -> DewVal_DewVal_merge/2
  CdsnProbltyMgr_Init/1(DewVal_DewVal_write) -> [DewVal_DewVal_merge_Tag1]
  [DewVal_DewVal_merge_Tag1] -> DewVal_DewVal_merge/1
  CdsnProbltyMgr_Runnable/1 -> CdsnProbltyMgr_Runnable_sys/Trigger

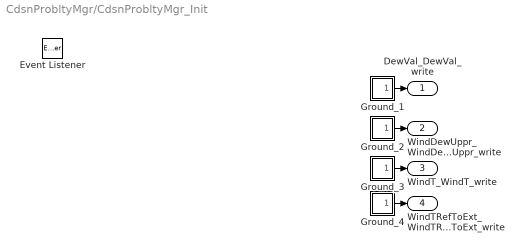
== system CdsnProbltyMgr/CdsnProbltyMgr_Init ==
  Event Listener : EventListener  EventName=reset
  Ground_1 : Subsystem
  Ground_2 : Subsystem
  Ground_3 : Subsystem
  Ground_4 : Subsystem
  DewVal_DewVal_write : Outport
  WindDewUppr_WindDewTUppr_write : Outport  Port=2
  WindT_WindT_write : Outport  Port=3
  WindTRefToExt_WindTRefToExt_write : Outport  Port=4
  wires:
  Ground_1/1(Out1) -> DewVal_DewVal_write/1
  Ground_2/1(Out1) -> WindDewUppr_WindDewTUppr_write/1
  Ground_3/1(Out1) -> WindT_WindT_write/1
  Ground_4/1(Out1) -> WindTRefToExt_WindTRefToExt_write/1
